simulink model "wlan_phy_rx_pmd"  (Simulink 7.8)
settings: Solver=VariableStepDiscrete  SolverMode=SingleTasking  StartTime=0.0  StopTime=rx_sim_time  RelTol=1e-3
PostLoadFcn:
  | wlan_phy_rx_init
InitFcn:
  | wlan_phy_rx_init
StartFcn:
  | wlan_phy_rx_init
values from neighbouring files (symbols this model uses):
  barker_seq20 = 0.95 * circshift(barker_seq20, [0 4]) ./ max(abs(barker_seq20))    [wlan_phy_rx_init.m]
  bit_scrambler_lfsr_bytes = sum(bit_scrambler_lfsr_bytes .* repmat(2.^[0:7], 127, 1),2)    [wlan_phy_rx_init.m]
  deinterleave_ROM = [deinterleave_ROM interleave_11n_64QAM zeros(1, 512-length(interleave_11n_64QAM))]    [wlan_phy_rx_init.m]
  longCorr_coef_i = [3*real(longCorr_coef)]    [wlan_phy_rx_init.m]
  longCorr_coef_q = [3*imag(longCorr_coef)]    [wlan_phy_rx_init.m]
  MAX_CP_LEN = 32    [wlan_phy_rx_init.m]
  MAX_NCBPS = NCBPS_11n_64QAM    (= 312)    [wlan_phy_rx_init.m]
  MAX_NUM_BYTES = 2^16-1    (= 65535)    [wlan_phy_rx_init.m]
  MAX_NUM_SC = 64    [wlan_phy_rx_init.m]
  MAX_NUM_SYMS = 600    [wlan_phy_rx_init.m]
  NCBPS_11a_BPSK = 48    [wlan_phy_rx_init.m]
  NDBPS_11a_BPSK12 = 24    [wlan_phy_rx_init.m]
  PHY_SIGNAL_MIN_LEN = 14    [wlan_phy_rx_init.m]
  preFFT_sampBuff_numSamps = 4*MAX_NUM_SC    (= 256)    [wlan_phy_rx_init.m]
  rx_sim_sig_samp_time = 8    [wlan_phy_rx_init.m]
  scr_ind_rev = zeros(1,128)    [wlan_phy_rx_init.m]
undefined symbols (not defined in the file or in its folder): gcbh, mcs_rom_11ag, mcs_rom_11n, xlBlockLoadCallback, xlGetNormalizedPeriod, xlipgetpartsetting
libraries: xbsIndex_r4 x3862, simulink x1, xbsEDKLib_r4 x1
(part 4 of 23)

== system wlan_phy_rx_pmd/Sync & Antenna Sel ==
  Rx I : Inport
  Rx Q : Inport  Port=2
  IQ Valid : Inport  Port=3
  RSSI : Inport  Port=4
  "Reset " : Inport  Port=5
  ADC/Corr : Scope  NumInputPorts=10
      settings: TimeRange=16508  YMin=0.95~-1~-1~0.95~-1~-1~-1~-1~-1~-1  YMax=1.05~1~1~1.05~1~1~1~1~1~1  SaveName=ScopeData34
  Antenna Selection : Subsystem
  Debug  Outputs : Subsystem
  Gateway Out{,1..4} (5 alike) : xbsIndex_r4/Gateway Out  inherit_from_input=off  hdl_port=off  timing_constraint=None  locs_specified=off  LOCs={}  UseAsDAC=off  DACChannel='1'  block_type=gatewayout
  LTS Correlation : Subsystem
  PHY CCA : Subsystem
  Pkt Det : Subsystem
  Pkt Det AGC Ctrl : Subsystem
  Resets : Subsystem
  " Pkt Det DSSS" : Outport
  " Rx I" : Outport  Port=2
  " Rx Q" : Outport  Port=3
  " IQ Valid" : Outport  Port=4
  " Pkt Det OFDM" : Outport  Port=5
  LTS Sync : Outport  Port=6
  wires:
  LTS Correlation/1(Det) -> LTS Sync/1, PHY CCA/1(LTS Sync), Debug  Outputs/1(LTS)
  LTS Correlation/2(Timeout) -> PHY CCA/2(LTS Timeout), Resets/3(LTS Timeout), Debug  Outputs/2(LTS Timeout), Gateway Out2/1
  Gateway Out2/1 -> ADC/Corr/3  "LTS TO"
  Gateway Out1/1 -> ADC/Corr/2  "Pkt Done"
  Gateway Out/1 -> ADC/Corr/1  "Pkt Det OFDM"
  Pkt Det/1(Det OFDM) -> Antenna Selection/1(Pkt Det), LTS Correlation/5(En), PHY CCA/4(Det OFDM), Debug  Outputs/3(Det OFDM), Gateway Out/1, " Pkt Det OFDM"/1, Pkt Det AGC Ctrl/1(Det OFDM)
  Resets/1(" OFDM Reset") -> Antenna Selection/6(Reset), LTS Correlation/6(Reset), PHY CCA/6(Reset OFDM), Gateway Out1/1, Pkt Det/1(Reset OFDM)
  IQ Valid/1 -> Pkt Det/5(IQ Valid), Antenna Selection/4(IQ Valid)
  RSSI/1 -> Pkt Det/6(RSSI Vec), Antenna Selection/5(RSSI Vec), PHY CCA/5(Avg RSSI Vec)
  Rx I/1 -> Pkt Det/3(Rx I Vec), Antenna Selection/2(Rx I Vec)
  Rx Q/1 -> Pkt Det/4(Rx Q Vec), Antenna Selection/3(Rx Q Vec)
  Antenna Selection/1(Rx I) -> " Rx I"/1, LTS Correlation/1(rx_I)
  Antenna Selection/2(" Rx Q") -> " Rx Q"/1, LTS Correlation/2(rx_Q)
  Antenna Selection/3(" IQ Valid") -> " IQ Valid"/1, LTS Correlation/3(rx_IQ_valid), PHY CCA/3(IQ Valid)
  Antenna Selection/4(RSSI) -> LTS Correlation/4(RSSI), Gateway Out3/1  "Avg RSSI"
  Gateway Out3/1 -> ADC/Corr/9
  Pkt Det/2(Det DSSS) -> Gateway Out4/1, " Pkt Det DSSS"/1, Pkt Det AGC Ctrl/2(Det DSSS), Debug  Outputs/4(Det DSSS), PHY CCA/7(Det DSSS)
  Gateway Out4/1 -> ADC/Corr/4  "Pkt Det DSSS"
  Resets/2(DSSS Reset) -> Pkt Det/2(Reset DSSS), PHY CCA/8(Reset DSSS)
  [DSSS_RX_RESET] -> Resets/2("DSSS Reset ")
  "Reset "/1 -> Resets/1(OFDM Reset)

== system wlan_phy_rx_pmd/CFO & Samp Buffer/CFO Correction ==
  note: DDS requires >1 cycle EN early to get setup
      | Use gloabl reset for this
      | Safe, since DDS output is ignored when
      | it's not being used, so bogus outputs
      | between packets is no problem
      |
      | DDS updates once per input sample
      | Since the FFT only consumes 64 samples
      | per OFDM symbol (ignoring the CP),
      | the DDS keeps running after each FFT
      | frame is loaded. This is fine, since the FFT is
      | configured for non-streaming (it ignores its
      | I/Q inputs while computing a transform)
  "I " : Inport
  "Q " : Inport  Port=2
  DDS Run : Inport  Port=3
  Valid : Inport  Port=4
  tlast : Inport  Port=5
  CFO Est : Inport  Port=6
  CFO Corr : Scope  NumInputPorts=8
      settings: TimeRange=35000  YMin=0~-0.4~0~-1~0~0~-0.4~0  YMax=1~0.4~1~2~1~1~0.4~1  SaveName=ScopeData3
  "Complex Multiplier 5.0 " : xbsIndex_r4/Complex Multiplier 5.0   hasatlast=off  hasatuser=on  atuserwidth=1  hasbtlast=off  hasbtuser=off  btuserwidth=1  multtype=Use_Mults  optimizegoal=Performance  flowcontrol=NonBlocking  outputwidth=16  roundmode=Truncate  hasctrltlast=off  hasctrltuser=off  ctrltuserwidth=1  outtlastbehv=Null  latencyconfig=Manual  minimumlatency=2  aclken=off  aresetn=off  trim_axipin_name=on  aportwidth=63  bportwidth=63  ip_name=Complex Multiplier  ip_version=5.0  dsptool_ready=true  ipcore_usecache=true  ipcore_useipmodelcache=true  ipcore_verbose=false  ipcore_latency_parameter='minimumlatency'  wrapper_available=true  port_translation_map={ 'aclken' => 'en', 'aresetn' => 'rst'}  structural_sim=false  block_type=cmpy_v5_0
  Constant1 : xbsIndex_r4/Constant  const=1  gui_display_data_type=Boolean  arith_type=Boolean  n_bits=16  bin_pt=14  preci_type=Single  exp_width=8  frac_width=24  explicit_period=off  period=8  equ=P=C  opselect=C  inp2=PCIN>>17  opr=+  inp1=P  carry=CIN  dbl_ovrd=off  block_type=constant
  Constant2 : xbsIndex_r4/Constant  const=1  gui_display_data_type=Fixed-point  arith_type=Signed (2's comp)  n_bits=16  bin_pt=15  preci_type=Single  exp_width=8  frac_width=24  explicit_period=on  period=1  equ=P=C  opselect=C  inp2=PCIN>>17  opr=+  inp1=P  carry=CIN  dbl_ovrd=off  block_type=constant
  Constant3 : xbsIndex_r4/Constant  const=0  gui_display_data_type=Fixed-point  arith_type=Signed (2's comp)  n_bits=16  bin_pt=15  preci_type=Single  exp_width=8  frac_width=24  explicit_period=on  period=1  equ=P=C  opselect=C  inp2=PCIN>>17  opr=+  inp1=P  carry=CIN  dbl_ovrd=off  block_type=constant
  Constant5 : xbsIndex_r4/Constant  const=1  gui_display_data_type=Boolean  arith_type=Boolean  n_bits=16  bin_pt=14  preci_type=Single  exp_width=8  frac_width=24  explicit_period=off  period=8  equ=P=C  opselect=C  inp2=PCIN>>17  opr=+  inp1=P  carry=CIN  dbl_ovrd=off  block_type=constant
  Convert1 : xbsIndex_r4/Convert  gui_display_data_type=Fixed-point  arith_type="Signed  (2's comp)"  n_bits=16  bin_pt=15  float_type=Single  exp_bits=8  fraction_bits=24  quantization=Truncate  overflow=Wrap  en=off  latency=0  dbl_ovrd=off  pipeline=off  block_type=convert
  Convert2 : xbsIndex_r4/Convert  gui_display_data_type=Fixed-point  arith_type="Signed  (2's comp)"  n_bits=16  bin_pt=15  float_type=Single  exp_bits=8  fraction_bits=24  quantization=Truncate  overflow=Wrap  en=off  latency=0  dbl_ovrd=off  pipeline=off  block_type=convert
  Convert3 : xbsIndex_r4/Convert  gui_display_data_type=Fixed-point  arith_type="Signed  (2's comp)"  n_bits=26  bin_pt=26  float_type=Single  exp_bits=8  fraction_bits=24  quantization=Truncate  overflow=Flag as error  en=off  latency=0  dbl_ovrd=off  pipeline=off  block_type=convert
  Convert4 : xbsIndex_r4/Convert  gui_display_data_type=Fixed-point  arith_type=Unsigned  n_bits=1  bin_pt=0  float_type=Single  exp_bits=8  fraction_bits=24  quantization=Truncate  overflow=Flag as error  en=off  latency=0  dbl_ovrd=off  pipeline=off  block_type=convert
  Convert5 : xbsIndex_r4/Convert  gui_display_data_type=Boolean  arith_type=Boolean  n_bits=1  bin_pt=0  float_type=Single  exp_bits=8  fraction_bits=24  quantization=Truncate  overflow=Flag as error  en=off  latency=0  dbl_ovrd=off  pipeline=off  block_type=convert
  DDS Compiler 4.0 : xbsIndex_r4/DDS Compiler 4.0   partspresent=Phase_Generator_and_SIN_COS_LUT  dds_clock_rate=160  channels=1  parameter_entry=Hardware_Parameters  spurious_free_dynamic_range=36  frequency_resolution=0.4  noise_shaping=None  phase_width=26  output_width=16  output_selection=Sine_and_Cosine  negative_sine=off  negative_cosine=off  amplitude_mode=Full_Range  memory_type=Auto  optimization_goal=Auto  dsp48_use=Minimal  latency_configuration=Auto  latency=6  has_phase_out=off  sclr_pin=off  clock_enable=on  rfd=off  rdy=on  channel_pin=off  explicit_period=off  period=1  phase_increment=Programmable  output_frequency1=0  output_frequency2=0  output_frequency3=0  output_frequency4=0  output_frequency5=0  output_frequency6=0  output_frequency7=0  output_frequency8=0  output_frequency9=0  output_frequency10=0  output_frequency11=0  output_frequency12=0  output_frequency13=0  output_frequency14=0  output_frequency15=0  output_frequency16=0  pinc1='0'  pinc2='0'  pinc3='0'  pinc4='0'  pinc5='0'  pinc6='0'  pinc7='0'  pinc8='0'  pinc9='0'  pinc10='0'  pinc11='0'  pinc12='0'  pinc13='0'  pinc14='0'  pinc15='0'  pinc16='0'  phase_offset=None  phase_offset_angles1=0  phase_offset_angles2=0  phase_offset_angles3=0  phase_offset_angles4=0  phase_offset_angles5=0  phase_offset_angles6=0  phase_offset_angles7=0  phase_offset_angles8=0  phase_offset_angles9=0  phase_offset_angles10=0  phase_offset_angles11=0  phase_offset_angles12=0  phase_offset_angles13=0  phase_offset_angles14=0  phase_offset_angles15=0  phase_offset_angles16=0  poff1='0'  poff2='0'  poff3='0'  poff4='0'  poff5='0'  poff6='0'  poff7='0'  poff8='0'  poff9='0'  poff10='0'  poff11='0'  poff12='0'  poff13='0'  poff14='0'  poff15='0'  poff16='0'  por_mode=false  gui_behaviour=Sysgen  ip_name=DDS Compiler  ip_version=4.0  dsptool_ready=true  wrapper_available=true  port_translation_map={ 'ce' => 'en', 'sclr' => 'rst' }  ipcore_xco_need_fpga_part=true  ipcore_fpga_part=xlipgetpartsetting(gcb, {'virtex4', 'xc4vsx35', '-10', 'ff668'})  ipcore_usecache=true  ipcore_useipmodelcache=true  ipcore_verbose=false  block_type=dds_compiler_v4_0
  Gateway Out{1..7,17} (8 alike) : xbsIndex_r4/Gateway Out  inherit_from_input=off  hdl_port=off  timing_constraint=None  locs_specified=off  LOCs={}  UseAsDAC=off  DACChannel='1'  block_type=gatewayout
  Logical2 : xbsIndex_r4/Logical  logical_function=OR  inputs=3  en=off  latency=0  precision=Full  arith_type=Unsigned  n_bits=16  bin_pt=0  align_bp=on  dbl_ovrd=off  block_type=logical
  Sim Reset : Subsystem
  valid : Outport
  I : Outport  Port=2
  Q : Outport  Port=3
  " tlast" : Outport  Port=4
  wires:
  Constant1/1 -> DDS Compiler 4.0/1
  CFO Est/1 -> Convert3/1
  DDS Run/1 -> Logical2/1
  "Complex Multiplier 5.0 "/2 -> Convert2/1, Gateway Out6/1
  "Complex Multiplier 5.0 "/3 -> Convert1/1
  "I "/1 -> "Complex Multiplier 5.0 "/3, Gateway Out1/1
  "Q "/1 -> "Complex Multiplier 5.0 "/2
  Gateway Out17/1 -> CFO Corr/1
  Gateway Out1/1 -> CFO Corr/2
  Gateway Out2/1 -> CFO Corr/3
  Gateway Out3/1 -> CFO Corr/4
  Gateway Out4/1 -> CFO Corr/5
  DDS Compiler 4.0/2 -> "Complex Multiplier 5.0 "/6, Gateway Out3/1
  Valid/1 -> "Complex Multiplier 5.0 "/1, Gateway Out2/1
  Convert1/1 -> I/1
  Convert2/1 -> Q/1
  "Complex Multiplier 5.0 "/1 -> valid/1, Gateway Out5/1
  DDS Compiler 4.0/3 -> "Complex Multiplier 5.0 "/7
  Logical2/1 -> DDS Compiler 4.0/3, Gateway Out17/1
  [RX_GLOBAL_RESET] -> Logical2/2
  Convert3/1 -> DDS Compiler 4.0/2
  Constant5/1 -> "Complex Multiplier 5.0 "/5
  tlast/1 -> Convert4/1
  "Complex Multiplier 5.0 "/4 -> Gateway Out7/1, Convert5/1
  Gateway Out5/1 -> CFO Corr/6
  Gateway Out6/1 -> CFO Corr/7
  Gateway Out7/1 -> CFO Corr/8
  Convert4/1 -> "Complex Multiplier 5.0 "/4, Gateway Out4/1
  Convert5/1 -> " tlast"/1
  Sim Reset/1(Out1) -> Logical2/3

== system wlan_phy_rx_pmd/CFO & Samp Buffer/CFO Estimation ==
  note: (1/2) for normalization of phase
      | (1/64) for number of summed elements
  LTS_Sync : Inport
  rx_I : Inport  Port=2
  rx_Q : Inport  Port=3
  rx_valid : Inport  Port=4
  CFO Est : Scope  NumInputPorts=10
      settings: TimeRange=10000  YMin=-1~-1~-0.25~-1~-10~-2.5~-1~0~0~-1  YMax=1~1~2~2~50~15~1~1~0.001~1
  Conj Mult : Subsystem
  Convert2 : xbsIndex_r4/Convert  gui_display_data_type=Fixed-point  arith_type="Signed  (2's comp)"  n_bits=21  bin_pt=13  float_type=Single  exp_bits=8  fraction_bits=24  quantization=Truncate  overflow=Flag as error  en=off  latency=0  dbl_ovrd=off  pipeline=off  block_type=convert
  Delay : xbsIndex_r4/Delay  rst=off  infoeditControl=Selection of Reset will increase slice count due to use of real FFs and instead of SRLs  en=off  latency=8  dbl_ovrd=off  reg_retiming=off  block_type=delay
  Delay1 : xbsIndex_r4/Delay  rst=off  infoeditControl=Selection of Reset will increase slice count due to use of real FFs and instead of SRLs  en=on  latency=64  dbl_ovrd=off  reg_retiming=off  block_type=delay
      settings: xl_area=[7 14 0 14 0 0 0]  block_version=VER_STRING_GOES_HERE
  Delay2 : xbsIndex_r4/Delay  rst=off  infoeditControl=Selection of Reset will increase slice count due to use of real FFs and instead of SRLs  en=on  latency=64  dbl_ovrd=off  reg_retiming=off  block_type=delay
      settings: xl_area=[7 14 0 14 0 0 0]  block_version=VER_STRING_GOES_HERE
  Delay3 : xbsIndex_r4/Delay  rst=off  infoeditControl=Selection of Reset will increase slice count due to use of real FFs and instead of SRLs  en=on  latency=60  dbl_ovrd=off  reg_retiming=off  block_type=delay
      settings: xl_area=[7 14 0 14 0 0 0]  block_version=VER_STRING_GOES_HERE
  Gateway Out{,1..8} (9 alike) : xbsIndex_r4/Gateway Out  inherit_from_input=off  hdl_port=off  timing_constraint=None  locs_specified=off  LOCs={}  UseAsDAC=off  DACChannel='1'  block_type=gatewayout
  Phase : Subsystem
  Posedge3 : Subsystem
  Register : xbsIndex_r4/Register  init=0  rst=on  en=on  dbl_ovrd=off  block_type=register
  Running Sum : Subsystem
  Running Sum1 : Subsystem
  S-R Latch : Subsystem
  Scale : xbsIndex_r4/Scale  scale_factor=-7  dbl_ovrd=off  block_type=scale
      settings: block_version=10.1.2
  CFO : Outport
  wires:
  Gateway Out5/1 -> CFO Est/6  "Sum Q"
  Gateway Out4/1 -> CFO Est/5  "Sum I"
  Gateway Out3/1 -> CFO Est/4  "Conj Prod Q"
  Gateway Out2/1 -> CFO Est/3  "Conj Prod I"
  Gateway Out1/1 -> CFO Est/2  "ADC Q"
  Gateway Out/1 -> CFO Est/1  "ADC I"
  rx_valid/1 -> Conj Mult/1(Valid), Delay2/2, Delay1/2, Delay3/2
  rx_I/1 -> Conj Mult/2(A I), Delay2/1, Gateway Out/1
  rx_Q/1 -> Conj Mult/3(A Q), Delay1/1, Gateway Out1/1
  Delay2/1 -> Conj Mult/4(B I)
  Delay1/1 -> Conj Mult/5(B Q)
  Conj Mult/2(" I") -> Running Sum/2(D), Gateway Out2/1
  Conj Mult/1(" Valid") -> Running Sum/1(En), Running Sum1/1(En)
  Conj Mult/3(" Q") -> Running Sum1/2(D), Gateway Out3/1
  Running Sum/1(Valid) -> Phase/1(Valid)
  Phase/2(Phase) -> Gateway Out6/1, Register/1
  Gateway Out6/1 -> CFO Est/7  "Phase(Sum)"
  Register/1 -> Convert2/1
  Convert2/1 -> Scale/1
  Scale/1 -> CFO/1, Gateway Out8/1
  Gateway Out7/1 -> CFO Est/8  "LTS Sync"
  LTS_Sync/1 -> S-R Latch/1(S)
  Gateway Out8/1 -> CFO Est/9  "CFO Est Out"
  ? -> CFO Est/10
  Delay3/1 -> Posedge3/1(D)
  S-R Latch/1(Q) -> Delay/1, Delay3/1
  Delay/1 -> S-R Latch/2(R)
  Posedge3/1(Q) -> Gateway Out7/1, Register/3
  Running Sum/2(Sum) -> Phase/2(Re), Gateway Out4/1
  Running Sum1/2(Sum) -> Phase/3(Im), Gateway Out5/1
  [regRx_BYPASS_CFO_EST] -> Register/2

== system wlan_phy_rx_pmd/CFO & Samp Buffer/FIFO ==
  note: AND not strictly necessary, since FFT
      | only honors tlast when tvalid is asserted.
      | Explicit AND here makes scopes
      | much easier to understand.
  note: This FIFO aids in synchronizing sample generation
      | and feeding the FFT. It would be possible to design
      | the sample buffer control logic not to require
      | this FIFO, but then the logic would need tweaking
      | if the FFT timing or sample processing timing
      | ever change. This FIFO isolates those blocks,
      | at the cost of a little memory and 1 clock cyle latency
      | (assuming the FFT consumes samples faster than
      | they're received)
  data tvalid : Inport
  I : Inport  Port=2
  Q : Inport  Port=3
  data tlast : Inport  Port=4
  FFT tready : Inport  Port=5
  AXI FIFO : xbsIndex_r4/AXI FIFO  input_depth_axis=128  actual_fifo_depth_label=130  enable_tdata=on  enable_tdest=off  enable_tstrb=off  enable_tready=on  enable_tid=off  enable_tuser=off  enable_tkeep=off  enable_tlast=on  has_aresetn=off  enable_data_counts_axis=on  fifo_implementation_type_axis=Common Clock Block RAM  trim_axipin_name=on  programmable_full_type_axis=Full  full_threshold_assert_value_axis=0  programmable_empty_type_axis=Empty  empty_threshold_assert_value_axis=1022  block_type=axi_fifo
  FIFO : Scope  NumInputPorts=9
      settings: TimeRange=14580  YMin=0.95~-1~-1~-1~0.95~-1~-1~-1~-1  YMax=1.05~1~1~1~1.05~1~1~1~1  SaveName=ScopeData20
  Gateway Out{1..6,8..9} (8 alike) : xbsIndex_r4/Gateway Out  inherit_from_input=off  hdl_port=off  timing_constraint=None  locs_specified=off  LOCs={}  UseAsDAC=off  DACChannel='1'  block_type=gatewayout
  I/Q Concat : Subsystem
  I/Q Slice : Subsystem
  Logical2 : xbsIndex_r4/Logical  logical_function=AND  inputs=2  en=off  latency=0  precision=Full  arith_type=Unsigned  n_bits=16  bin_pt=0  align_bp=on  dbl_ovrd=off  block_type=logical
  FFT tvalid : Outport
  FFT tdata_re : Outport  Port=2
  FFT tdata_im : Outport  Port=3
  FFT tlast : Outport  Port=4
  FIFO tready : Outport  Port=5
  wires:
  AXI FIFO/2 -> I/Q Slice/1(IQ)
  AXI FIFO/1 -> FFT tvalid/1, Gateway Out1/1, Logical2/1
  AXI FIFO/3 -> Logical2/2
  Gateway Out8/1 -> FIFO/1  "FFT ready"
  I/Q Slice/1(I) -> FFT tdata_re/1
  I/Q Slice/2(Q) -> FFT tdata_im/1, Gateway Out2/1
  FFT tready/1 -> Gateway Out8/1, AXI FIFO/1
  data tvalid/1 -> Gateway Out5/1, AXI FIFO/2
  data tlast/1 -> Gateway Out6/1, AXI FIFO/4
  AXI FIFO/4 -> FIFO tready/1, Gateway Out4/1
  Gateway Out1/1 -> FIFO/2  "FIFO out valid"
  Gateway Out2/1 -> FIFO/3  "Q out"
  Gateway Out3/1 -> FIFO/4  "tlast out"
  Gateway Out4/1 -> FIFO/5  "FIFO ready"
  Gateway Out5/1 -> FIFO/6  "valid in"
  Gateway Out6/1 -> FIFO/7  "tlast in"
  Logical2/1 -> FFT tlast/1, Gateway Out3/1
  I/1 -> I/Q Concat/1(I)
  Q/1 -> I/Q Concat/2(Q)
  I/Q Concat/1(IQ) -> AXI FIFO/3
  ? -> FIFO/8  "Reset"
  Gateway Out9/1 -> FIFO/9  "FIFO Occ"
  AXI FIFO/5 -> Gateway Out9/1

== system wlan_phy_rx_pmd/CFO & Samp Buffer/Samp Buffer ==
  note: Circular sample buffer records all incoming I/Q samples post pkt det
      | Samples are unloaded from the buffer after LTS sync and fed into
      | the FFT (via CFO correction and a FIFO)
      |
      | This buffer is required because 802.11 uses the same preamble samples
      | (the LTS) for sample-level synchronization and CFO estimation. The
      | received LTS samples must be saved and CFO-corrected before the
      | FFT to avoid degrading the channel estimates.
  Pkt Det : Inport
  Pkt Done : Inport  Port=2
  rx I : Inport  Port=3
  rx Q : Inport  Port=4
  IQ valid : Inport  Port=5
  LTS Sync 2 : Inport  Port=6
  FIFO tready : Inport  Port=7
  Circular Sample Buffer : xbsIndex_r4/Dual Port RAM  depth=preFFT_sampBuff_numSamps  initVector=1 * repmat([0:63], 1, 4)  distributed_mem=Block RAM  init_a=0  init_b=0  rst_a=off  rst_b=off  en_a=off  en_b=off  latency=1  write_mode_A=Read After Write  write_mode_B=Read After Write  dbl_ovrd=off  optimize=Area  block_type=dpram
  Concat : Subsystem
  Constant3 : xbsIndex_r4/Constant  const=0  gui_display_data_type=Fixed-point  arith_type=Unsigned  n_bits=32  bin_pt=0  preci_type=Single  exp_width=8  frac_width=24  explicit_period=off  period=8  equ=P=C  opselect=C  inp2=PCIN>>17  opr=+  inp1=P  carry=CIN  dbl_ovrd=off  block_type=constant
  Constant4 : xbsIndex_r4/Constant  const=0  gui_display_data_type=Boolean  arith_type=Boolean  n_bits=6  bin_pt=0  preci_type=Single  exp_width=8  frac_width=24  explicit_period=off  period=8  equ=P=C  opselect=C  inp2=PCIN>>17  opr=+  inp1=P  carry=CIN  dbl_ovrd=off  block_type=constant
  Counter : xbsIndex_r4/Counter  cnt_type=Free Running  cnt_to=Inf  operation=Up  start_count=0  cnt_by_val=1  arith_type=Unsigned  n_bits=ceil(log2(preFFT_sampBuff_numSamps))  bin_pt=0  load_pin=off  rst=off  en=on  explicit_period=off  period=8  dbl_ovrd=off  use_behavioral_HDL=off  implementation=Fabric  block_type=counter
  Delay : xbsIndex_r4/Delay  rst=off  infoeditControl=Selection of Reset will increase slice count due to use of real FFs and instead of SRLs  en=off  latency=1  dbl_ovrd=off  reg_retiming=off  block_type=delay
  Delay2 : xbsIndex_r4/Delay  rst=off  infoeditControl=Selection of Reset will increase slice count due to use of real FFs and instead of SRLs  en=off  latency=1  dbl_ovrd=off  reg_retiming=off  block_type=delay
  FFT Load Ctrl : Subsystem
  FSM : Subsystem
  Gateway Out{1..8} (8 alike) : xbsIndex_r4/Gateway Out  inherit_from_input=off  hdl_port=off  timing_constraint=None  locs_specified=off  LOCs={}  UseAsDAC=off  DACChannel='1'  block_type=gatewayout
  Logical2 : xbsIndex_r4/Logical  logical_function=AND  inputs=2  en=off  latency=0  precision=Full  arith_type=Unsigned  n_bits=16  bin_pt=0  align_bp=on  dbl_ovrd=off  block_type=logical
  Posedge : Subsystem
  Posedge3 : Subsystem
  Register : xbsIndex_r4/Register  init=0  rst=off  en=off  dbl_ovrd=off  block_type=register
  Register1 : xbsIndex_r4/Register  init=0  rst=off  en=off  dbl_ovrd=off  block_type=register
  S-R Latch : Subsystem
  Scope : Scope  NumInputPorts=9
      settings: TimeRange=10000  YMin=-0.25~-0.25~0~0~0~-0.1~-0.1~-5~-5  YMax=0.2~0.2~300~300~4000~1.1~1.1~5~5  SaveName=ScopeData25
  Slice : Subsystem
  Terminator : Terminator
  dbg_payload : xbsIndex_r4/Gateway Out  inherit_from_input=off  hdl_port=on  timing_constraint=None  locs_specified=off  LOCs={}  UseAsDAC=off  DACChannel='1'  block_type=gatewayout
  I : Outport
  Q : Outport  Port=2
  Buff Rd : Outport  Port=3
  " IQ valid" : Outport  Port=4
  tlast : Outport  Port=5
  tag scope: OFDM_RX_RUNNING_DSSS_BLOCK=global
  wires:
  Concat/1(IQ) -> Circular Sample Buffer/2
  Circular Sample Buffer/1 -> Terminator/1
  FFT Load Ctrl/1(Rd Addr) -> Circular Sample Buffer/4, Gateway Out1/1
  Constant3/1 -> Circular Sample Buffer/5
  Constant4/1 -> Circular Sample Buffer/6
  Logical2/1 -> Posedge/1(D)
  Posedge/1(Q) -> FFT Load Ctrl/3(LTS Sync), FSM/2(LTS)
  FFT Load Ctrl/5(FFT Load Done) -> FSM/1(FFT Load Done), Gateway Out7/1
  FSM/3(FFT Data load) -> FFT Load Ctrl/6(FFT Load)
  Circular Sample Buffer/2 -> Slice/1(IQ)
  FSM/1(FFT skip sel) -> FFT Load Ctrl/4(Skip Sel)
  FFT Load Ctrl/3(FFT tvalid) -> Delay/1, Posedge3/1(D)
  FFT Load Ctrl/2(Reading) -> Delay2/1
  Pkt Done/1 -> FSM/3(Pkt Done), S-R Latch/2(R), Gateway Out4/1
  rx I/1 -> Concat/1(I), Gateway Out6/1
  rx Q/1 -> Concat/2(Q)
  IQ valid/1 -> Circular Sample Buffer/3, Counter/1
  Slice/1(I) -> I/1, Gateway Out5/1
  Slice/2(Q) -> Q/1
  FIFO tready/1 -> FFT Load Ctrl/2(data tready)
  Delay2/1 -> Buff Rd/1
  Delay/1 -> " IQ valid"/1, Gateway Out8/1
  FFT Load Ctrl/4(FFT tlast) -> tlast/1
  LTS Sync 2/1 -> Logical2/1
  Pkt Det/1 -> Logical2/2, Gateway Out3/1
  Gateway Out5/1 -> Scope/2  "FFT In I"
  Gateway Out1/1 -> Scope/3  "Rd Addr"
  Gateway Out2/1 -> Scope/4  "Wr Addr"
  Gateway Out3/1 -> Scope/5  "Pkt Det"
  Posedge3/1(Q) -> S-R Latch/1(S)
  S-R Latch/1(Q) -> Register/1, [OFDM_RX_RUNNING_DSSS_BLOCK]
  Gateway Out4/1 -> Scope/6  "Pkt Done"
  Gateway Out6/1 -> Scope/1  "ADC I"
  Register/1 -> Register1/1
  Register1/1 -> dbg_payload/1
  Gateway Out7/1 -> Scope/7  "FFT Load Done"
  Counter/1 -> Circular Sample Buffer/1, FFT Load Ctrl/1(Wr Addr), Gateway Out2/1
  FSM/2(FFT LTS load) -> FFT Load Ctrl/5(FFT LTS Load)
  Gateway Out8/1 -> Scope/9  "FFT In tvalid"

== system wlan_phy_rx_pmd/Chan Est & EQ/Chan Est ==
  x_re : Inport
  x_im : Inport  Port=2
  x_ind : Inport  Port=3
  x_valid : Inport  Port=4
  Sym Ind : Inport  Port=5
  Abs : Abs
  Abs1 : Abs
  Chan Est Offload : Subsystem
  Control : Subsystem
  Delay{1..4,7} (5 alike) : xbsIndex_r4/Delay  rst=off  infoeditControl=Selection of Reset will increase slice count due to use of real FFs and instead of SRLs  en=off  latency=2  dbl_ovrd=off  reg_retiming=off  block_type=delay
      settings: xl_area=[0, 0, 0, 0, 0, 0, 0]  block_version=VER_STRING_GOES_HERE
  Gateway Out{1..6,8} (7 alike) : xbsIndex_r4/Gateway Out  inherit_from_input=off  hdl_port=off  timing_constraint=None  locs_specified=off  LOCs={}  UseAsDAC=off  DACChannel='1'  block_type=gatewayout
  H Est Outputs : Scope  NumInputPorts=9
      settings: TimeRange=4000  YMin=-0.5~-1~-0.6~-0.5~0~0~0~0~-5  YMax=0.5~0.5~0.4~1~80~1~3~0.8~5  SaveName=ScopeData16
  I Estimate Buffer : Subsystem
  Inverter : xbsIndex_r4/Inverter  en=off  latency=0  dbl_ovrd=off  block_type=inv
  Logical1 : xbsIndex_r4/Logical  logical_function=AND  inputs=2  en=off  latency=0  precision=Full  arith_type=Unsigned  n_bits=16  bin_pt=0  align_bp=on  dbl_ovrd=off  block_type=logical
  Logical2 : xbsIndex_r4/Logical  logical_function=AND  inputs=2  en=off  latency=0  precision=Full  arith_type=Unsigned  n_bits=16  bin_pt=0  align_bp=on  dbl_ovrd=off  block_type=logical
  Mult by LTF : Subsystem
  Q Estimate Buffer : Subsystem
  Real-Imag to Complex : RealImagToComplex
  Real-Imag to Complex1 : RealImagToComplex
  To Float : Subsystem
  " x_I" : Outport
  " x_Q" : Outport  Port=2
  H_I : Outport  Port=3
  H_Q : Outport  Port=4
  " valid" : Outport  Port=5
  " x_ind" : Outport  Port=6
  " sym_ind" : Outport  Port=7
  wires:
  Logical2/1 -> I Estimate Buffer/3(Est_WE), Q Estimate Buffer/3(Est_WE)
  x_valid/1 -> Logical2/1, Logical1/1
  Sym Ind/1 -> Delay2/1, Control/1(Sym Ind)
  Control/1(Est Overwrite) -> I Estimate Buffer/4(First Sym), Q Estimate Buffer/4(First Sym)
  Control/2(Est WrEn) -> Logical2/2
  Delay7/1 -> Gateway Out4/1, To Float/5(x_ind), Chan Est Offload/1(x_ind)
  Delay1/1 -> Gateway Out5/1, To Float/6(x_valid), Chan Est Offload/4(x_valid)
  Logical1/1 -> Delay1/1
  Inverter/1 -> Logical1/2
  I Estimate Buffer/1(h[n]) -> Gateway Out2/1, To Float/3("H_I "), Chan Est Offload/2(x_I)
  Q Estimate Buffer/1(h[n]) -> Gateway Out3/1, To Float/4("H_Q "), Chan Est Offload/3(x_Q)
  Delay2/1 -> To Float/7(sym_ind), Chan Est Offload/5(sym_ind), Gateway Out6/1
  Delay3/1 -> To Float/1("x_I ")
  Delay4/1 -> To Float/2("x_Q ")
  Gateway Out8/1 -> Real-Imag to Complex/1, H Est Outputs/1  "x_I"
  Gateway Out1/1 -> Real-Imag to Complex/2, H Est Outputs/2  "x_Q"
  Gateway Out2/1 -> Real-Imag to Complex1/1, H Est Outputs/3  "H_I"
  Gateway Out3/1 -> Real-Imag to Complex1/2, H Est Outputs/4  "H_Q"
  Gateway Out4/1 -> H Est Outputs/5  "SC Ind"
  Gateway Out5/1 -> H Est Outputs/7  "Valid Out"
  x_ind/1 -> Delay7/1, I Estimate Buffer/2(Est_Addr), Q Estimate Buffer/2(Est_Addr), Mult by LTF/3(x_ind)
  x_re/1 -> Delay3/1, Mult by LTF/1(x_re)
  x_im/1 -> Delay4/1, Mult by LTF/2(x_im)
  Mult by LTF/2(H_im) -> Gateway Out1/1, Q Estimate Buffer/1(Est_Data)
  Mult by LTF/1(H_re) -> I Estimate Buffer/1(Est_Data), Gateway Out8/1
  To Float/1(" x_I") -> " x_I"/1
  To Float/2(" x_Q") -> " x_Q"/1
  To Float/3(H_I) -> H_I/1
  To Float/4(" H_Q") -> H_Q/1
  To Float/5(" x_ind") -> " x_ind"/1
  To Float/6(" x_valid") -> " valid"/1
  To Float/7(" sym_ind") -> " sym_ind"/1
  Abs/1 -> H Est Outputs/9
  Real-Imag to Complex/1 -> Abs/1
  Real-Imag to Complex1/1 -> Abs1/1
  Abs1/1 -> H Est Outputs/8
  Gateway Out6/1 -> H Est Outputs/6  "Sym Ind"
  Control/3(Scale) -> I Estimate Buffer/5(Scale), Q Estimate Buffer/5(Scale)
  Control/4(Sym Invalid) -> Inverter/1

== system wlan_phy_rx_pmd/Chan Est & EQ/Debug Outputs ==
  note: Only capture data and pilot subcarriers
      | for debug outputs and chipscope
  I : Inport
  Q : Inport  Port=2
  valid : Inport  Port=3
  x_ind : Inport  Port=4
  4x Buffer : Subsystem
  Constant : xbsIndex_r4/Constant  const=0  gui_display_data_type=Fixed-point  arith_type=Unsigned  n_bits=1  bin_pt=0  preci_type=Single  exp_width=8  frac_width=24  explicit_period=off  period=1  equ=P=C  opselect=C  inp2=PCIN>>17  opr=+  inp1=P  carry=CIN  dbl_ovrd=off  block_type=constant
  Constant1 : xbsIndex_r4/Constant  const=27  gui_display_data_type=Fixed-point  arith_type=Unsigned  n_bits=5  bin_pt=0  preci_type=Single  exp_width=8  frac_width=24  explicit_period=off  period=1  equ=P=C  opselect=C  inp2=PCIN>>17  opr=+  inp1=P  carry=CIN  dbl_ovrd=off  block_type=constant
  Constant2 : xbsIndex_r4/Constant  const=37  gui_display_data_type=Fixed-point  arith_type=Unsigned  n_bits=6  bin_pt=0  preci_type=Single  exp_width=8  frac_width=24  explicit_period=off  period=1  equ=P=C  opselect=C  inp2=PCIN>>17  opr=+  inp1=P  carry=CIN  dbl_ovrd=off  block_type=constant
  Debug EQ IQ : Scope  NumInputPorts=7
      settings: YMin=-1.1~-1~-1.1~-1~-5~-5~-5  YMax=1.1~1~1.2~1~5~5~5  SaveName=ScopeData13
  Delay : xbsIndex_r4/Delay  rst=off  infoeditControl=Selection of Reset will increase slice count due to use of real FFs and instead of SRLs  en=off  latency=1  dbl_ovrd=off  reg_retiming=off  block_type=delay
  Delay1 : xbsIndex_r4/Delay  rst=off  infoeditControl=Selection of Reset will increase slice count due to use of real FFs and instead of SRLs  en=off  latency=1  dbl_ovrd=off  reg_retiming=off  block_type=delay
  Gateway Out12 : xbsIndex_r4/Gateway Out  inherit_from_input=off  hdl_port=off  timing_constraint=None  locs_specified=off  LOCs={}  UseAsDAC=off  DACChannel='1'  block_type=gatewayout
  Gateway Out13 : xbsIndex_r4/Gateway Out  inherit_from_input=off  hdl_port=off  timing_constraint=None  locs_specified=off  LOCs={}  UseAsDAC=off  DACChannel='1'  block_type=gatewayout
  Gateway Out8 : xbsIndex_r4/Gateway Out  inherit_from_input=off  hdl_port=off  timing_constraint=None  locs_specified=off  LOCs={}  UseAsDAC=off  DACChannel='1'  block_type=gatewayout
  Inverter1 : xbsIndex_r4/Inverter  en=off  latency=0  dbl_ovrd=off  block_type=inv
  Logical : xbsIndex_r4/Logical  logical_function=AND  inputs=3  en=off  latency=0  precision=Full  arith_type=Unsigned  n_bits=16  bin_pt=0  align_bp=on  dbl_ovrd=off  block_type=logical
  Logical1 : xbsIndex_r4/Logical  logical_function=OR  inputs=2  en=off  latency=0  precision=Full  arith_type=Unsigned  n_bits=16  bin_pt=0  align_bp=on  dbl_ovrd=off  block_type=logical
  Real-Imag to Complex : RealImagToComplex
  Register{,1..2,7..8} (5 alike) : xbsIndex_r4/Register  init=0  rst=off  en=off  dbl_ovrd=off  block_type=register
  Register{3..6} (4 alike) : xbsIndex_r4/Register  init=0  rst=on  en=off  dbl_ovrd=off  block_type=register
  Relational : xbsIndex_r4/Relational  mode=a!=b  en=off  latency=0  dbl_ovrd=off  block_type=relational
  Relational1 : xbsIndex_r4/Relational  mode=a<b  en=off  latency=0  dbl_ovrd=off  block_type=relational
  Relational2 : xbsIndex_r4/Relational  mode=a>b  en=off  latency=0  dbl_ovrd=off  block_type=relational
  To FP1 : xbsIndex_r4/Convert  gui_display_data_type=Fixed-point  arith_type="Signed  (2's comp)"  n_bits=14  bin_pt=13  float_type=Single  exp_bits=8  fraction_bits=24  quantization=Truncate  overflow=Saturate  en=off  latency=0  dbl_ovrd=off  pipeline=off  block_type=convert
  To FP4 : xbsIndex_r4/Convert  gui_display_data_type=Fixed-point  arith_type="Signed  (2's comp)"  n_bits=14  bin_pt=13  float_type=Single  exp_bits=8  fraction_bits=24  quantization=Truncate  overflow=Saturate  en=off  latency=0  dbl_ovrd=off  pipeline=off  block_type=convert
  To Workspace : ToWorkspace  VariableName=eq_out  SampleTime=-1  SaveFormat=Array
  To Workspace1 : ToWorkspace  VariableName=eq_out_valid  SampleTime=-1  SaveFormat=Array
  XY Graph : simulink/Sinks/XY Graph  xmin=-1.2  xmax=1.2  ymin=-1.2  ymax=1.2  st=1
  dbg_EQ_I : xbsIndex_r4/Gateway Out  inherit_from_input=off  hdl_port=on  timing_constraint=None  locs_specified=off  LOCs={}  UseAsDAC=off  DACChannel='1'  block_type=gatewayout
  dbg_EQ_Q : xbsIndex_r4/Gateway Out  inherit_from_input=off  hdl_port=on  timing_constraint=None  locs_specified=off  LOCs={}  UseAsDAC=off  DACChannel='1'  block_type=gatewayout
  dbg_EQ_Q{1..4} (4 alike) : xbsIndex_r4/Gateway Out  inherit_from_input=off  hdl_port=off  timing_constraint=None  locs_specified=off  LOCs={}  UseAsDAC=off  DACChannel='1'  block_type=gatewayout
  tag scope: CS_EQ_I=global, CS_EQ_Q=global, CS_EQ_Valid=global
  wires:
  Inverter1/1 -> Register3/2, Register6/2
  Logical/1 -> Inverter1/1, Delay/1, Gateway Out8/1, 4x Buffer/3(Vin)
  Register2/1 -> To FP1/1, Register6/1, Gateway Out13/1
  Register1/1 -> To FP4/1, Register3/1, Gateway Out12/1
  x_ind/1 -> Register/1
  Register/1 -> Relational/1, Relational1/1, Relational2/1
  Constant/1 -> Relational/2
  Relational/1 -> Logical/2
  I/1 -> Register1/1
  Q/1 -> Register2/1
  Constant1/1 -> Relational1/2
  Constant2/1 -> Relational2/2
  Relational1/1 -> Logical1/1
  Relational2/1 -> Logical1/2
  Logical1/1 -> Logical/3
  Register3/1 -> [CS_EQ_I], dbg_EQ_Q3/1
  Register6/1 -> [CS_EQ_Q], dbg_EQ_Q1/1
  Delay/1 -> [CS_EQ_Valid], dbg_EQ_Q2/1
  valid/1 -> Delay1/1
  Delay1/1 -> Logical/1
  Register7/1 -> dbg_EQ_I/1
  Register8/1 -> dbg_EQ_Q/1
  dbg_EQ_I/1 -> XY Graph/1, Debug EQ IQ/3
  dbg_EQ_Q/1 -> XY Graph/2, Debug EQ IQ/4
  dbg_EQ_Q3/1 -> Debug EQ IQ/5
  dbg_EQ_Q1/1 -> Debug EQ IQ/6
  dbg_EQ_Q2/1 -> Debug EQ IQ/7
  Gateway Out12/1 -> Real-Imag to Complex/1
  Gateway Out13/1 -> Real-Imag to Complex/2
  Real-Imag to Complex/1 -> To Workspace/1
  Gateway Out8/1 -> To Workspace1/1
  To FP4/1 -> 4x Buffer/1(I)
  To FP1/1 -> 4x Buffer/2(Q)
  Register4/1 -> Register7/1
  4x Buffer/1(" I") -> Register4/1
  4x Buffer/3(#Vout) -> Register4/2, Register5/2, dbg_EQ_Q4/1
  4x Buffer/2(" Q") -> Register5/1
  Register5/1 -> Register8/1
  dbg_EQ_Q4/1 -> Debug EQ IQ/2

== system wlan_phy_rx_pmd/Chan Est & EQ/Equalizer, Pilot Processing & PHY Mode Det ==
  note: PHY Mode affects many subsystems - use Goto/From
      | tags to avoid messy routing of these control signals
  x_I : Inport
  x_Q : Inport  Port=2
  H_I : Inport  Port=3
  H_Q : Inport  Port=4
  x_valid : Inport  Port=5
  x_ind : Inport  Port=6
  Sym ind : Inport  Port=7
  Convert : xbsIndex_r4/Convert  gui_display_data_type=Fixed-point  arith_type="Signed  (2's comp)"  n_bits=16  bin_pt=14  float_type=Single  exp_bits=8  fraction_bits=24  quantization="Round  (unbiased: +/- Inf)"  overflow=Saturate  en=off  latency=1  dbl_ovrd=off  pipeline=off  block_type=convert
  Convert1 : xbsIndex_r4/Convert  gui_display_data_type=Fixed-point  arith_type="Signed  (2's comp)"  n_bits=16  bin_pt=14  float_type=Single  exp_bits=8  fraction_bits=24  quantization="Round  (unbiased: +/- Inf)"  overflow=Saturate  en=off  latency=1  dbl_ovrd=off  pipeline=off  block_type=convert
  Delay{1,3..4,8,10} (5 alike) : xbsIndex_r4/Delay  rst=off  infoeditControl=Selection of Reset will increase slice count due to use of real FFs and instead of SRLs  en=off  latency=62  dbl_ovrd=off  reg_retiming=off  block_type=delay
  Delay12 : xbsIndex_r4/Delay  rst=off  infoeditControl=Selection of Reset will increase slice count due to use of real FFs and instead of SRLs  en=off  latency=23  dbl_ovrd=off  reg_retiming=off  block_type=delay
  Delay14 : xbsIndex_r4/Delay  rst=off  infoeditControl=Selection of Reset will increase slice count due to use of real FFs and instead of SRLs  en=off  latency=23  dbl_ovrd=off  reg_retiming=off  block_type=delay
  Delay17 : xbsIndex_r4/Delay  rst=off  infoeditControl=Selection of Reset will increase slice count due to use of real FFs and instead of SRLs  en=off  latency=23  dbl_ovrd=off  reg_retiming=off  block_type=delay
  Delay2 : xbsIndex_r4/Delay  rst=off  infoeditControl=Selection of Reset will increase slice count due to use of real FFs and instead of SRLs  en=off  latency=8  dbl_ovrd=off  reg_retiming=off  block_type=delay
  Delay6 : xbsIndex_r4/Delay  rst=off  infoeditControl=Selection of Reset will increase slice count due to use of real FFs and instead of SRLs  en=off  latency=8  dbl_ovrd=off  reg_retiming=off  block_type=delay
  Delay7 : xbsIndex_r4/Delay  rst=off  infoeditControl=Selection of Reset will increase slice count due to use of real FFs and instead of SRLs  en=off  latency=8  dbl_ovrd=off  reg_retiming=off  block_type=delay
  Divide : xbsIndex_r4/Divide  flow_control=NonBlocking  axi_optimize_goal=Resources  algorithm_type=High_Radix  infoedit1=Specify output fraction-width for Fixed-point division.  fractional_width=16  latency=21  rate=1  has_a_tlast=off  has_a_tuser=off  has_b_tlast=off  has_b_tuser=off  en=off  has_result_tready=off  result_tlast_behv=Pass_A_TLAST  has_underflow=off  has_overflow=off  has_invalid_op=off  has_divide_by_zero=off  fpo_op_type=mergeddivide  block_type=divide
      settings: infoedit=Blocking: Wait for data on all inputs, allow back-pressure.<br>NonBlocking: operate every cycle in which all inputs are valid, no back-pressure.
  Divide1 : xbsIndex_r4/Divide  flow_control=NonBlocking  axi_optimize_goal=Resources  algorithm_type=High_Radix  infoedit1=Specify output fraction-width for Fixed-point division.  fractional_width=16  latency=21  rate=1  has_a_tlast=off  has_a_tuser=off  has_b_tlast=off  has_b_tuser=off  en=off  has_result_tready=off  result_tlast_behv=Pass_A_TLAST  has_underflow=off  has_overflow=off  has_invalid_op=off  has_divide_by_zero=off  fpo_op_type=mergeddivide  block_type=divide
      settings: infoedit=Blocking: Wait for data on all inputs, allow back-pressure.<br>NonBlocking: operate every cycle in which all inputs are valid, no back-pressure.
  EQ : Scope  NumInputPorts=8
      settings: TimeRange=6000  YMin=-1~-1~-1~-1~-1~-2.1~-2.1~0  YMax=1~1~1~1~1~-1.9~-1.9~1  SaveName=ScopeData9
  Gateway Out{,1..7} (8 alike) : xbsIndex_r4/Gateway Out  inherit_from_input=off  hdl_port=off  timing_constraint=None  locs_specified=off  LOCs={}  UseAsDAC=off  DACChannel='1'  block_type=gatewayout
  Logical4 : xbsIndex_r4/Logical  logical_function=OR  inputs=2  en=off  latency=0  precision=Full  arith_type=Unsigned  n_bits=16  bin_pt=0  align_bp=on  dbl_ovrd=off  block_type=logical
  Mag : Subsystem
  PHY Mode Det : Subsystem
  Phase Err Calc : Subsystem
  Register3 : xbsIndex_r4/Register  init=0  rst=off  en=off  dbl_ovrd=off  block_type=register
  Register4 : xbsIndex_r4/Register  init=0  rst=off  en=off  dbl_ovrd=off  block_type=register
  Register5 : xbsIndex_r4/Register  init=0  rst=off  en=off  dbl_ovrd=off  block_type=register
  a*conj(b) : Subsystem
  " x_I" : Outport
  " x_Q" : Outport  Port=2
  " x_valid" : Outport  Port=3
  phase err : Outport  Port=4
  " x_ind" : Outport  Port=5
  " sym ind" : Outport  Port=6
  tag scope: RX_PHY_MODE_11AC=global, RX_PHY_MODE_11AG=global, RX_PHY_MODE_11N=global, RX_PHY_MODE_11N_AC=global
  wires:
  x_ind/1 -> Gateway Out5/1, Delay2/1
  Sym ind/1 -> Gateway Out6/1, Delay7/1
  x_I/1 -> a*conj(b)/1(A_I), Gateway Out/1
  x_Q/1 -> a*conj(b)/2(A_Q), Gateway Out1/1
  H_I/1 -> a*conj(b)/3(B_I), Mag/1(I)
  H_Q/1 -> a*conj(b)/4(B_Q), Mag/2(Q)
  x_valid/1 -> Gateway Out4/1, Delay6/1
  Phase Err Calc/1(Sym Phase Err) -> phase err/1
  Delay7/1 -> Phase Err Calc/5(sym_ind), Delay17/1
  a*conj(b)/1(P_I) -> Phase Err Calc/1(CP_I), Register4/1
  a*conj(b)/2(P_Q) -> Phase Err Calc/2(CP_Q), Register3/1
  Delay2/1 -> Phase Err Calc/4(x_ind), Delay14/1
  Mag/1(M) -> Register5/1
  Divide/1 -> Convert/1
  Divide1/1 -> Convert1/1
  Convert/1 -> Delay8/1, PHY Mode Det/1(x_I)
  Convert1/1 -> Delay10/1, PHY Mode Det/2(x_Q)
  Register3/1 -> Divide1/1
  Register4/1 -> Divide/1
  Delay12/1 -> Delay4/1, PHY Mode Det/3(x_valid)
  Delay14/1 -> Delay1/1
  Delay17/1 -> Delay3/1, PHY Mode Det/4(Sym ind)
  Delay6/1 -> Phase Err Calc/3(x_valid), Delay12/1
  Gateway Out6/1 -> EQ/5  "Sym Ind"
  Gateway Out5/1 -> EQ/4  "X Ind"
  Gateway Out4/1 -> EQ/3  "X Valid"
  Gateway Out2/1 -> EQ/7  "EQ Q"
  Gateway Out1/1 -> EQ/2  "X_Q"
  Gateway Out/1 -> EQ/1  "X_I"
  Gateway Out7/1 -> EQ/6  "EQ I"
  Gateway Out3/1 -> EQ/8  "EQ Valid"
  Register5/1 -> Divide/2, Divide1/2
  PHY Mode Det/3(11a/g) -> [RX_PHY_MODE_11AG]
  PHY Mode Det/2(11n) -> [RX_PHY_MODE_11N], Logical4/2
  PHY Mode Det/1(11ac) -> [RX_PHY_MODE_11AC], Logical4/1
  Logical4/1 -> [RX_PHY_MODE_11N_AC]
  Delay8/1 -> " x_I"/1, Gateway Out7/1
  Delay10/1 -> " x_Q"/1, Gateway Out2/1
  Delay4/1 -> " x_valid"/1, Gateway Out3/1
  Delay1/1 -> " x_ind"/1
  Delay3/1 -> " sym ind"/1

== system wlan_phy_rx_pmd/Chan Est & EQ/Phase Correction ==
  x_I : Inport
  x_Q : Inport  Port=2
  x_valid : Inport  Port=3
  Phase : Inport  Port=4
  "x_ind " : Inport  Port=5
  "sym_ind " : Inport  Port=6
  "CORDIC 5.0 " : xbsIndex_r4/CORDIC 5.0   functional_selection=Rotate  architectural_configuration=Parallel  pipelining_mode=Optimal  data_format=SignedFraction  phase_format=Scaled_Radians  input_width=16  output_width=18  round_mode=Truncate  iterations=0  precision=0  compensation_scaling=Embedded_Multiplier  coarse_rotation=on  aclken=off  aresetn=off  out_tready=off  cartesian_has_tuser=off  cartesian_has_tlast=off  cartesian_tuser_width=1  phase_has_tuser=off  phase_has_tlast=off  phase_tuser_width=1  out_tlast_behv=Null  flow_control=NonBlocking  optimize_goal=Performance  trim_axipin_name=on  ip_name=CORDIC  ip_version=5.0  dsptool_ready=true  ipcore_usecache=true  ipcore_useipmodelcache=true  wrapper_available=true  port_translation_map={ 'aclken' => 'en', 'aresetn' => 'rst' }  block_type=cordic_v5_0
      settings: infoedit=CORDIC 5.0
  Constant1 : xbsIndex_r4/Constant  const=1  gui_display_data_type=Boolean  arith_type=Boolean  n_bits=16  bin_pt=13  preci_type=Single  exp_width=8  frac_width=24  explicit_period=on  period=1  equ=P=C  opselect=C  inp2=PCIN>>17  opr=+  inp1=P  carry=CIN  dbl_ovrd=off  block_type=constant
  Convert : xbsIndex_r4/Convert  gui_display_data_type=Fixed-point  arith_type="Signed  (2's comp)"  n_bits=16  bin_pt=13  float_type=Single  exp_bits=8  fraction_bits=24  quantization=Truncate  overflow=Flag as error  en=off  latency=0  dbl_ovrd=off  pipeline=off  block_type=convert
  Delay1 : xbsIndex_r4/Delay  rst=off  infoeditControl=Selection of Reset will increase slice count due to use of real FFs and instead of SRLs  en=off  latency=24  dbl_ovrd=off  reg_retiming=off  block_type=delay
  Delay18 : xbsIndex_r4/Delay  rst=off  infoeditControl=Selection of Reset will increase slice count due to use of real FFs and instead of SRLs  en=off  latency=24  dbl_ovrd=off  reg_retiming=off  block_type=delay
  Gateway Out{5,8..13} (7 alike) : xbsIndex_r4/Gateway Out  inherit_from_input=off  hdl_port=off  timing_constraint=None  locs_specified=off  LOCs={}  UseAsDAC=off  DACChannel='1'  block_type=gatewayout
  Negate : xbsIndex_r4/Negate  precision=Full  arith_type=Unsigned  n_bits=16  bin_pt=14  quantization=Truncate  overflow=Wrap  en=off  latency=0  dbl_ovrd=off  block_type=negate
  Phase Correct : Scope  NumInputPorts=8
      settings: TimeRange=10000  YMin=-1~-2.1~-2.1~-1~0.304413~-1~0.930648~-0.840211  YMax=1~-1.9~-1.9~1~0.336457~1~1.02861~-0.760191  SaveName=ScopeData14
  " x_I" : Outport
  " x_Q" : Outport  Port=2
  " x_valid" : Outport  Port=3
  x_ind : Outport  Port=4
  " sym ind" : Outport  Port=5
  tag scope: CS_Phase_Err=global
  wires:
  Delay18/1 -> " sym ind"/1
  "sym_ind "/1 -> Delay18/1
  Delay1/1 -> x_ind/1
  "x_ind "/1 -> Delay1/1
  Negate/1 -> Convert/1
  Constant1/1 -> "CORDIC 5.0 "/4
  "CORDIC 5.0 "/3 -> " x_I"/1, Gateway Out9/1
  "CORDIC 5.0 "/2 -> " x_Q"/1, Gateway Out10/1
  "CORDIC 5.0 "/1 -> " x_valid"/1, Gateway Out13/1
  Convert/1 -> "CORDIC 5.0 "/5
  x_valid/1 -> "CORDIC 5.0 "/1, Gateway Out5/1
  x_Q/1 -> "CORDIC 5.0 "/2, Gateway Out11/1
  x_I/1 -> "CORDIC 5.0 "/3, Gateway Out8/1
  Gateway Out10/1 -> Phase Correct/8  "Q Out"
  Gateway Out13/1 -> Phase Correct/6  "Valid Out"
  Gateway Out5/1 -> Phase Correct/1  "X_IQ Valid"
  Gateway Out8/1 -> Phase Correct/2  "X_I"
  Gateway Out9/1 -> Phase Correct/7  "I Out"
  Gateway Out11/1 -> Phase Correct/3  "X_Q"
  Gateway Out12/1 -> Phase Correct/5  "Phase"
  Phase/1 -> Gateway Out12/1, [CS_Phase_Err], Negate/1

== system wlan_phy_rx_pmd/DSSS Rx/Demod ==
  Start : Inport
  I : Inport  Port=2
  Q : Inport  Port=3
  Bit-to-Byte : Subsystem
  DBPSK Demod : Subsystem
  DSSS Rx : Scope  NumInputPorts=7
      settings: TimeRange=56900  YMin=-1~-1~0.95~0~47.5~0~0  YMax=1~1~1.05~1~80~1~250
  De-spread : Subsystem
  Descramble : Subsystem
  Gateway Out{1..7} (7 alike) : xbsIndex_r4/Gateway Out  inherit_from_input=off  hdl_port=off  timing_constraint=None  locs_specified=off  LOCs={}  UseAsDAC=off  DACChannel='1'  block_type=gatewayout
  Header CRC : Subsystem
  Register8 : xbsIndex_r4/Register  init=0  rst=off  en=on  dbl_ovrd=off  block_type=register
  Sync : Subsystem
  Byte : Outport
  Byte Valid : Outport  Port=2
  Byte Ind : Outport  Port=3
  CRC Calc : Outport  Port=4
  tag scope: CS_DSSS_BIT_VALID=global
  wires:
  Q/1 -> De-spread/2(Q), Gateway Out3/1
  Start/1 -> De-spread/3(Sym Start), Gateway Out6/1
  I/1 -> De-spread/1(I), Gateway Out2/1
  De-spread/1(IQ Valid) -> DBPSK Demod/1(IQ Valid)
  De-spread/2(" I") -> DBPSK Demod/2(I)
  De-spread/3(" Q") -> DBPSK Demod/3(Q)
  DBPSK Demod/1(bit) -> Descramble/1(Bit)
  DBPSK Demod/2(bit valid) -> Descramble/2(Bit Valid)
  Descramble/1(" Bit") -> Register8/1, Sync/1(Bit)
  Descramble/2(" Bit Valid") -> Sync/2(Bit Valid), Register8/2, [CS_DSSS_BIT_VALID]
  Gateway Out2/1 -> DSSS Rx/1
  Gateway Out3/1 -> DSSS Rx/2
  Gateway Out6/1 -> DSSS Rx/3
  Gateway Out7/1 -> DSSS Rx/4
  Gateway Out1/1 -> DSSS Rx/5
  Gateway Out4/1 -> DSSS Rx/6
  Gateway Out5/1 -> DSSS Rx/7
  Sync/1(Payload Bit) -> Bit-to-Byte/1(Bit), Header CRC/1(Bit)
  Sync/2(" Bit Valid") -> Bit-to-Byte/2(Bit Valid), Header CRC/2(Bit Valid)
  Bit-to-Byte/3(Byte Ind) -> Byte Ind/1, Gateway Out1/1
  Bit-to-Byte/1(Byte) -> Byte/1, Gateway Out5/1
  Bit-to-Byte/2(Byte Valid) -> Byte Valid/1, Gateway Out4/1
  Register8/1 -> Gateway Out7/1
  Header CRC/1(CRC Calc) -> CRC Calc/1

== system wlan_phy_rx_pmd/DSSS Rx/Reset Gen ==
  note: Extend the DSSS reset event long enough to flush
      | the DSSS PHY pipeline and to reduce the
      | chance of spurious double-detections
  Reset : Inport
  Constant1 : xbsIndex_r4/Constant  const=127  gui_display_data_type=Fixed-point  arith_type=Unsigned  n_bits=7  bin_pt=0  preci_type=Single  exp_width=8  frac_width=24  explicit_period=off  period=1  equ=P=C  opselect=C  inp2=PCIN>>17  opr=+  inp1=P  carry=CIN  dbl_ovrd=off  block_type=constant
      settings: block_version=VER_STRING_GOES_HERE
  Counter1 : xbsIndex_r4/Counter  cnt_type=Free Running  cnt_to=Inf  operation=Up  start_count=0  cnt_by_val=1  arith_type=Unsigned  n_bits=7  bin_pt=0  load_pin=off  rst=on  en=on  explicit_period=off  period=1  dbl_ovrd=off  use_behavioral_HDL=off  implementation=Fabric  block_type=counter
  Down Sample3 : xbsIndex_r4/Down Sample  sample_ratio=8  sample_phase="Last Value of Frame  (most efficient)"  en=off  latency=1  dbl_ovrd=off  block_type=dsamp
  Inverter2 : xbsIndex_r4/Inverter  en=off  latency=0  dbl_ovrd=off  block_type=inv
  Logical1 : xbsIndex_r4/Logical  logical_function=OR  inputs=2  en=off  latency=0  precision=Full  arith_type=Unsigned  n_bits=8  bin_pt=2  align_bp=on  dbl_ovrd=off  block_type=logical
      settings: xl_area=[1 0 0 1 0 0 0]  block_version=VER_STRING_GOES_HERE
  Logical2 : xbsIndex_r4/Logical  logical_function=OR  inputs=2  en=off  latency=0  precision=Full  arith_type=Unsigned  n_bits=8  bin_pt=2  align_bp=on  dbl_ovrd=off  block_type=logical
      settings: xl_area=[1 0 0 1 0 0 0]  block_version=VER_STRING_GOES_HERE
  Posedge : Subsystem
  Relational1 : xbsIndex_r4/Relational  mode=a=b  en=off  latency=0  dbl_ovrd=off  block_type=relational
  S-R Latch : Subsystem
  S-R Latch2 : Subsystem
  negedge : Subsystem
  DSSS Rx Reset : Outport
  wires:
  Posedge/1(Q) -> S-R Latch/1(S), Logical1/1
  S-R Latch/1(Q) -> Logical2/2
  Counter1/1 -> Relational1/1
  Constant1/1 -> Relational1/2
  negedge/1(Q) -> S-R Latch2/1(S)
  S-R Latch2/1(Q) -> Counter1/2, Inverter2/1
  Relational1/1 -> S-R Latch2/2(R), S-R Latch/2(R)
  Inverter2/1 -> Logical1/2
  Reset/1 -> Posedge/1(D), negedge/1(D), Logical2/1
  Down Sample3/1 -> DSSS Rx Reset/1
  Logical1/1 -> Counter1/1
  Logical2/1 -> Down Sample3/1

== system wlan_phy_rx_pmd/DSSS Rx/S-R Latch1 ==
  S : Inport
  R : Inport  Port=2
  Constant1 : xbsIndex_r4/Constant  const=1  gui_display_data_type=Boolean  arith_type=Boolean  n_bits=1  bin_pt=0  preci_type=Single  exp_width=8  frac_width=24  explicit_period=off  period=1  equ=P=C  opselect=C  inp2=PCIN>>17  opr=+  inp1=P  carry=CIN  dbl_ovrd=off  block_type=constant
      settings: block_version=VER_STRING_GOES_HERE
  Convert1 : xbsIndex_r4/Convert  gui_display_data_type=Boolean  arith_type=Boolean  n_bits=1  bin_pt=0  float_type=Single  exp_bits=8  fraction_bits=24  quantization=Truncate  overflow=Wrap  en=off  latency=0  dbl_ovrd=off  pipeline=off  block_type=convert
  Convert2 : xbsIndex_r4/Convert  gui_display_data_type=Boolean  arith_type=Boolean  n_bits=1  bin_pt=0  float_type=Single  exp_bits=8  fraction_bits=24  quantization=Truncate  overflow=Wrap  en=off  latency=0  dbl_ovrd=off  pipeline=off  block_type=convert
  Register2 : xbsIndex_r4/Register  init=0  rst=on  en=on  dbl_ovrd=off  block_type=register
      settings: xl_area=[1 1 0 0 0 0 0]  block_version=VER_STRING_GOES_HERE
  Q : Outport
  wires:
  S/1 -> Convert2/1
  Register2/1 -> Q/1
  R/1 -> Convert1/1
  Constant1/1 -> Register2/1
  Convert1/1 -> Register2/2
  Convert2/1 -> Register2/3
